simulink model "ObsvrCmptmtT"  (R2022b)
settings: Solver=FixedStepDiscrete  StartTime=0.0  StopTime=10.0  FixedStep=1  RelTol=1e-3
settings Model: Creator=blue.ge  LastModifiedBy=blue.ge
settings DataIOCC: SaveFormat=StructureWithTime
settings OptimizationCC: DefaultParameterBehavior=Inlined  EnhancedBackFolding=on  PassReuseOutputArgsAs=Individual arguments  ZeroExternalMemoryAtStartup=off  ZeroInternalMemoryAtStartup=off  OptimizeBlockOrder=speed  DifferentSizesBufferReuse=on  OptimizationCustomize=off  EfficientTunableParamExpr=on
settings DebuggingCC: ParameterTunabilityLossMsg=error
settings ModelReferenceCC: ModelReferenceNumInstancesAllowed=Single
settings SFSimCC: MATLABDynamicMemAlloc=off
settings RTWCC: Description=AUTOSAR  SystemTargetFile=autosar.tlc  GenCodeOnly=on  TemplateMakefile=ert_default_tmf
settings CodeAppCC: ForceParamTrailComments=on  IgnoreCustomStorageClasses=off  OperatorAnnotations=on  SimulinkDataObjDesc=on  SFDataObjDesc=on  InsertBlockDesc=on
settings STFCustomTargetCC: TargetLangStandard=C99 (ISO)  MultiwordLength=256  ParMdlRefBuildCompliant=on  CompOptLevelCompliant=on  IncludeMdlTerminateFcn=off  CombineOutputUpdateFcns=on  SuppressErrorStatus=on  ERTCustomFileBanners=on  SupportNonFinite=off  SupportComplex=off  SupportContinuousTime=off  SupportNonInlinedSFcns=off  RemoveResetFunc=on  MATLABClassNameForMDSCustomization=Simulink.SoftwareTarget.ERTCustomization  AutosarCompliant=on  UseToolchainInfoCompliant=on  LookupTableObjectStructAxisOrder=2,1,3,4,...  LUTObjectStructOrderEvenSpacing=Size,Table,Breakpoints  SystemTargetFile=autosar.tlc
settings ERTTargetCC: TargetLangStandard=C99 (ISO)  ConcurrentExecutionCompliant=off  IncludeMdlTerminateFcn=off  SuppressErrorStatus=on  SupportNonFinite=off  SupportComplex=off  ModelStepFunctionPrototypeControlCompliant=off  CPPClassGenCompliant=off  AutosarCompliant=on  LookupTableObjectStructAxisOrder=2,1,3,4,...  LUTObjectStructOrderEvenSpacing=Size,Table,Breakpoints  InlinedParameterPlacement=NonHierarchical  ERTCustomFileTemplate=example_file_process.tlc

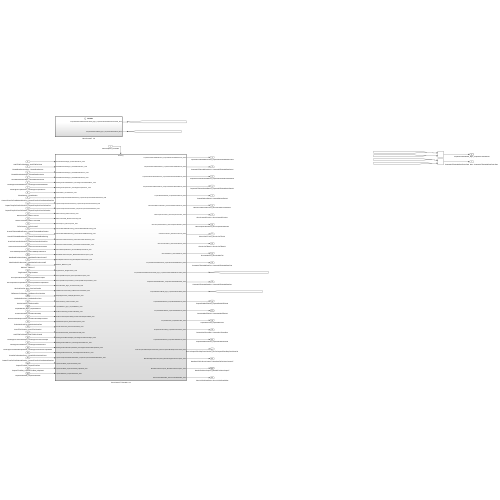
[thumbnail: Simulink's stored preview of the top-level diagram]
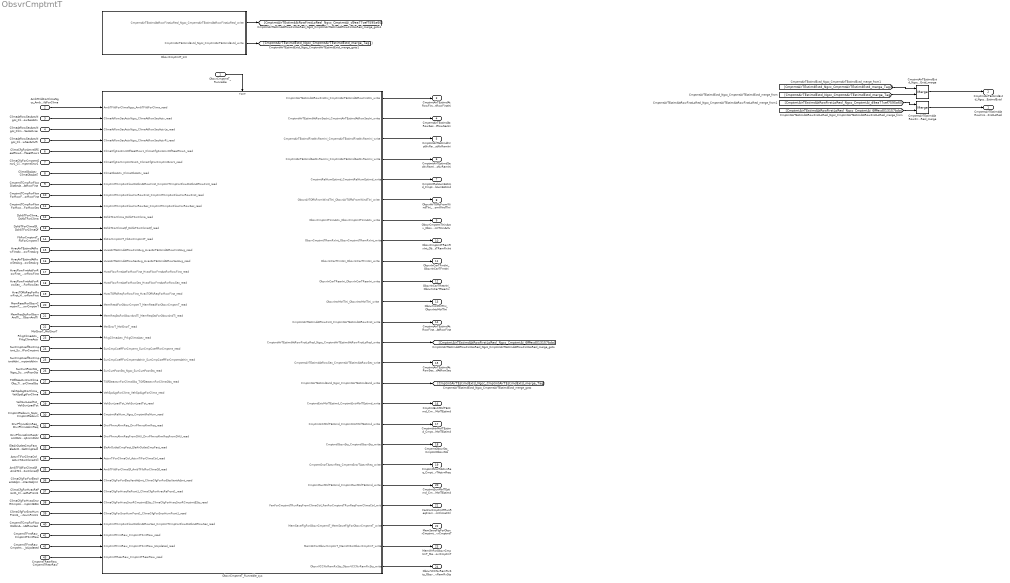
== system ObsvrCmptmtT ==
  ObsvrCmptmtT_Runnable : Inport  SampleTime=1  OutputFunctionCall=on
  AmbTFildForClimaNgcc_AmbTFildForClima : Inport  Port=2  OutDataTypeStr=T30  PortDimensions=1
  ClimaAtRowSecActvNgcc_ClimaAtRowSecActv : Inport  Port=3  OutDataTypeStr=Boolean  PortDimensions=1
  ClimaAtRowSecActvNgcc_ClimaAtRowSecActvLe : Inport  Port=4  OutDataTypeStr=Boolean  PortDimensions=1
  ClimaAtRowSecActvNgcc_ClimaAtRowSecActvRi : Inport  Port=5  OutDataTypeStr=Boolean  PortDimensions=1
  ClimaCfgForAmntOfSeatRow1_ClimaCfgForAmntOfSeatRow1 : Inport  Port=6  OutDataTypeStr=Enum: ClimaCfgForAmntOfSeatRow1  PortDimensions=1
  ClimaCfgForCmptmtSnsr1_ClimaCfgForCmptmtSnsr1 : Inport  Port=7  OutDataTypeStr=Enum: ClimaCfgForCmptmtSnsr1  PortDimensions=1
  ClimaObsActv_ClimaObsActv : Inport  Port=8  OutDataTypeStr=Boolean  PortDimensions=1
  CmptmtTCmpForFlowDistbnAtRowFirst_CmptmtTCmpForFlowDistbnAtRowFirst : Inport  Port=9  OutDataTypeStr=Fac30  PortDimensions=1
  CmptmtTCmpForFlowForRowFirst_CmptmtTCmpForFlowForRowFirst : Inport  Port=10  OutDataTypeStr=Fac30  PortDimensions=1
  CmptmtTCmpForFlowForRowSec_CmptmtTCmpForFlowForRowSec : Inport  Port=11  OutDataTypeStr=Fac30  PortDimensions=1
  DshbTForClima_DshbTForClima : Inport  Port=12  OutDataTypeStr=T30  PortDimensions=1
  DshbTForClimaQf_DshbTForClimaQf : Inport  Port=13  OutDataTypeStr=Enum: DshbTForClimaQf  PortDimensions=1
  FbForCmptmtT_FbForCmptmtT : Inport  Port=14  OutDataTypeStr=T30  PortDimensions=1
  HvacAirTEstimdAtRowFirstAvg_HvacAirTEstimdAtRowFirstAvg : Inport  Port=15  OutDataTypeStr=T30  PortDimensions=1
  HvacAirTEstimdAtRowSecAvg_HvacAirTEstimdAtRowSecAvg : Inport  Port=16  OutDataTypeStr=T30  PortDimensions=1
  HvacFlowFrntActForRowFirst_HvacFlowFrntActForRowFirst : Inport  Port=17  OutDataTypeStr=AirFlow30  PortDimensions=1
  HvacFlowFrntActForRowSec_HvacFlowFrntActForRowSec : Inport  Port=18  OutDataTypeStr=AirFlow30  PortDimensions=1
  HvacTOffsReqForRowFirst_HvacTOffsReqForRowFirst : Inport  Port=19  OutDataTypeStr=T30  PortDimensions=1
  MemReadForObsvrCmptmT_MemReadForObsvrCmptmT : Inport  Port=20  OutDataTypeStr=Bus: MemRecForObsvrCmptmT  PortDimensions=1  BusOutputAsStruct=on
  MemReqStsForObsvrAndTi_MemReqStsForObsvrAndTi : Inport  Port=21  OutDataTypeStr=Enum: MemReqSts  PortDimensions=1
  MtrlSnsrT_MtrlSnsrT : Inport  Port=22  OutDataTypeStr=Bus: MtrlSnsrT  PortDimensions=1  BusOutputAsStruct=on
  PrkgClimaActv_PrkgClimaActv : Inport  Port=23  OutDataTypeStr=Enum: PrkgClimaActv  PortDimensions=1
  SunCmpCoeffForCmptmt_SunCmpCoeffForCmptmt : Inport  Port=24  OutDataTypeStr=Fac30  PortDimensions=1
  SunCmpCoeffForCmptmtAtInin_SunCmpCoeffForCmptmtAtInin : Inport  Port=25  OutDataTypeStr=FacForSunCmpInin  PortDimensions=1
  SunCurtPosnSts_Ngcc_SunCurtPosnSts : Inport  Port=26  OutDataTypeStr=Enum: WinAndRoofAndCurtPosnTyp  PortDimensions=1
  TiOfDeactvnForClimaObs_TiOfDeactvnForClimaObs : Inport  Port=27  OutDataTypeStr=Ti33  PortDimensions=1
  VehSpdLgtForClima_VehSpdLgtForClima : Inport  Port=28  OutDataTypeStr=Bus: VehSpdLgtForClima  PortDimensions=1  BusOutputAsStruct=on
  VehSunLoadTot_VehSunLoadTot : Inport  Port=29  OutDataTypeStr=SunLoad30  PortDimensions=1
  CmptmtRelHum_Ngcc_CmptmtRelHum : Inport  Port=30  OutDataTypeStr=Bus: CmptmRelHum  PortDimensions=1  BusOutputAsStruct=on
  DrvrPfmncAlrmReq_DrvrPfmncAlrmReq : Inport  Port=31  OutDataTypeStr=Enum: DrvrPfmncWarnReq  PortDimensions=1
  DrvrPfmncAlrmReqFromDHU_DrvrPfmncAlrmReqFromDHU : Inport  Port=32  OutDataTypeStr=Enum: DrvrPfmncWarnReq  PortDimensions=1
  EleAirOutletCmpFact_EleAirOutletCmpFact : Inport  Port=33  OutDataTypeStr=VCCFac30  PortDimensions=1
  ActvnTiForClimaCtrl_ActvnTiForClimaCtrl : Inport  Port=34  OutDataTypeStr=Ti33  PortDimensions=1
  AmbTFildForClimaQf_AmbTFildForClimaQf : Inport  Port=35  OutDataTypeStr=Enum: GenQf1  PortDimensions=1
  ClimaCfgForForElecVentAdjmt_ClimaCfgForForElecVentAdjmt : Inport  Port=36  OutDataTypeStr=Boolean  PortDimensions=1
  ClimaCfgForHvacRePrsnt1_ClimaCfgForHvacRePrsnt1 : Inport  Port=37  OutDataTypeStr=Enum: CarCfgForHvacRe  PortDimensions=1
  ClimaCfgForHvacSnsrRCmptmtESts_ClimaCfgForHvacSnsrRCmptmtESts : Inport  Port=38  OutDataTypeStr=Enum: ClimaCfgForHvacSnsrRCmptmtESts  PortDimensions=1
  ClimaCfgForSnsrHumPrsnt1_ClimaCfgForSnsrHumPrsnt1 : Inport  Port=39  OutDataTypeStr=Boolean  PortDimensions=1
  CmptmtTCmpForFlowDistbnAtRowSec_CmptmtTCmpForFlowDistbnAtRowSec : Inport  Port=40  OutDataTypeStr=Fac30  PortDimensions=1
  CmptmtTFrntRaw_CmptmtTFrntRaw : Inport  Port=41  OutDataTypeStr=Bus: CmptmtTFrnt  PortDimensions=1  BusOutputAsStruct=on
  CmptmtTFrntRaw_CmptmtTFrntRaw_IsUpdated : Inport  Port=42  OutDataTypeStr=boolean  PortDimensions=1
  CmptmtTRearRaw_CmptmtTRearRaw : Inport  Port=43  OutDataTypeStr=Bus: CmptmtTFrnt  PortDimensions=1  BusOutputAsStruct=on
  CmptmtAirTEstimdAtRowFirstLoResl_Ngcc_CmptmtAirTEstimdAtRowFirstLoResl_merge : Merge
  CmptmtAirTEstimdExtd_Ngcc_CmptmtAirTEstimdExtd_merge : Merge
  ObsvrCmptmtT_Init : Subsystem
  ObsvrCmptmtT_Runnable_sys : Subsystem
  CmptmtAirTEstimdAtRowFirstLoResl_Ngcc_CmptmtAirTEstimdAtRowFirstLoResl : Outport  OutMin=-60  OutMax=125  OutDataTypeStr=TForCmptmt  PortDimensions=1
  CmptmtAirTEstimdExtd_Ngcc_CmptmtAirTEstimdExtd : Outport  Port=2  OutDataTypeStr=Bus: CmptmtAirTEstimdExtd  PortDimensions=1  BusOutputAsStruct=on
  CmptmtAirTEstimdAtRowFirstIni_CmptmtAirTEstimdAtRowFirstIni : Outport  Port=3  OutDataTypeStr=SInt8  PortDimensions=1
  CmptmtAirTEstimdAtRowSecIni_CmptmtAirTEstimdAtRowSecIni : Outport  Port=4  OutDataTypeStr=SInt8  PortDimensions=1
  CmptmtAirTEstimdFirstNvRamIni_CmptmtAirTEstimdFirstNvRamIni : Outport  Port=5  OutDataTypeStr=SInt8  PortDimensions=1
  CmptmtAirTEstimdSecNvRamIni_CmptmtAirTEstimdSecNvRamIni : Outport  Port=6  OutDataTypeStr=SInt8  PortDimensions=1
  CmptmtRelHumEstimd_CmptmtRelHumEstimd : Outport  Port=7  OutDataTypeStr=Perc7  PortDimensions=1
  ObsvrAirTOffsFromWindTIni_ObsvrAirTOffsFromWindTIni : Outport  Port=8  OutDataTypeStr=SInt8  PortDimensions=1
  ObsvrCmptmtTIninActv_ObsvrCmptmtTIninActv : Outport  Port=9  OutDataTypeStr=UInt8  PortDimensions=1
  ObsvrCmptmtTRamRxInt_ObsvrCmptmtTRamRxInt : Outport  Port=10  OutDataTypeStr=Enum: VCCNvRamRxSts  PortDimensions=1
  ObsvrInCarTFrntIni_ObsvrInCarTFrntIni : Outport  Port=11  OutDataTypeStr=SInt8  PortDimensions=1
  ObsvrInCarTRearIni_ObsvrInCarTRearIni : Outport  Port=12  OutDataTypeStr=SInt8  PortDimensions=1
  ObsvrIntrMtrlTIni_ObsvrIntrMtrlTIni : Outport  Port=13  OutDataTypeStr=SInt8  PortDimensions=1
  CmptmtAirTEstimdAtRowFirst_CmptmtAirTEstimdAtRowFirst : Outport  Port=14  OutDataTypeStr=T30  PortDimensions=1
  CmptmtAirTEstimdAtRowSec_CmptmtAirTEstimdAtRowSec : Outport  Port=15  OutDataTypeStr=T30  PortDimensions=1
  CmptmtExtrMtrlTEstimd_CmptmtExtrMtrlTEstimd : Outport  Port=16  OutDataTypeStr=T30  PortDimensions=1
  CmptmtIntrMtrlTEstimd_CmptmtIntrMtrlTEstimd : Outport  Port=17  OutDataTypeStr=Bus: MtrlSnsrT  PortDimensions=1  BusOutputAsStruct=on
  CmptmtObsvrSts_CmptmtObsvrSts : Outport  Port=18  OutDataTypeStr=Enum: ObsvrStsForCmptmt  PortDimensions=1
  CmptmtSnsrTActvnReq_CmptmtSnsrTActvnReq : Outport  Port=19  OutDataTypeStr=Enum: OnOff1  PortDimensions=1
  CmptmtSunMtrlTEstimd_CmptmtSunMtrlTEstimd : Outport  Port=20  OutDataTypeStr=T30  PortDimensions=1
  FanForCmptmtTRunReqFromClimaCtrl_FanForCmptmtTRunReqFromClimaCtrl : Outport  Port=21  OutDataTypeStr=Enum: OnOff1  PortDimensions=1
  MemSaveFlgForObsvrCmptmtT_MemSaveFlgForObsvrCmptmtT : Outport  Port=22  OutDataTypeStr=Boolean  PortDimensions=1
  MemWrForObsvrCmptmT_MemWrForObsvrCmptmT : Outport  Port=23  OutDataTypeStr=Bus: MemRecForObsvrCmptmT  PortDimensions=1  BusOutputAsStruct=on
  ObsvrVCCNvRamRxSts_ObsvrVCCNvRamRxSts : Outport  Port=24  OutDataTypeStr=Enum: VCCNvRamRxSts  PortDimensions=1
  wires:
  AmbTFildForClimaNgcc_AmbTFildForClima/1 -> ObsvrCmptmtT_Runnable_sys/1(AmbTFildForClimaNgcc_AmbTFildForClima_read)
  ClimaAtRowSecActvNgcc_ClimaAtRowSecActv/1 -> ObsvrCmptmtT_Runnable_sys/2(ClimaAtRowSecActvNgcc_ClimaAtRowSecActv_read)
  ClimaAtRowSecActvNgcc_ClimaAtRowSecActvLe/1 -> ObsvrCmptmtT_Runnable_sys/3(ClimaAtRowSecActvNgcc_ClimaAtRowSecActvLe_read)
  ClimaAtRowSecActvNgcc_ClimaAtRowSecActvRi/1 -> ObsvrCmptmtT_Runnable_sys/4(ClimaAtRowSecActvNgcc_ClimaAtRowSecActvRi_read)
  ClimaCfgForAmntOfSeatRow1_ClimaCfgForAmntOfSeatRow1/1 -> ObsvrCmptmtT_Runnable_sys/5(ClimaCfgForAmntOfSeatRow1_ClimaCfgForAmntOfSeatRow1_read)
  ClimaCfgForCmptmtSnsr1_ClimaCfgForCmptmtSnsr1/1 -> ObsvrCmptmtT_Runnable_sys/6(ClimaCfgForCmptmtSnsr1_ClimaCfgForCmptmtSnsr1_read)
  ClimaObsActv_ClimaObsActv/1 -> ObsvrCmptmtT_Runnable_sys/7(ClimaObsActv_ClimaObsActv_read)
  CmptmtTCmpForFlowDistbnAtRowFirst_CmptmtTCmpForFlowDistbnAtRowFirst/1 -> ObsvrCmptmtT_Runnable_sys/8(CmptmtTCmpForFlowDistbnAtRowFirst_CmptmtTCmpForFlowDistbnAtRowFirst_read)
  CmptmtTCmpForFlowForRowFirst_CmptmtTCmpForFlowForRowFirst/1 -> ObsvrCmptmtT_Runnable_sys/9(CmptmtTCmpForFlowForRowFirst_CmptmtTCmpForFlowForRowFirst_read)
  CmptmtTCmpForFlowForRowSec_CmptmtTCmpForFlowForRowSec/1 -> ObsvrCmptmtT_Runnable_sys/10(CmptmtTCmpForFlowForRowSec_CmptmtTCmpForFlowForRowSec_read)
  DshbTForClima_DshbTForClima/1 -> ObsvrCmptmtT_Runnable_sys/11(DshbTForClima_DshbTForClima_read)
  DshbTForClimaQf_DshbTForClimaQf/1 -> ObsvrCmptmtT_Runnable_sys/12(DshbTForClimaQf_DshbTForClimaQf_read)
  FbForCmptmtT_FbForCmptmtT/1 -> ObsvrCmptmtT_Runnable_sys/13(FbForCmptmtT_FbForCmptmtT_read)
  HvacAirTEstimdAtRowFirstAvg_HvacAirTEstimdAtRowFirstAvg/1 -> ObsvrCmptmtT_Runnable_sys/14(HvacAirTEstimdAtRowFirstAvg_HvacAirTEstimdAtRowFirstAvg_read)
  HvacAirTEstimdAtRowSecAvg_HvacAirTEstimdAtRowSecAvg/1 -> ObsvrCmptmtT_Runnable_sys/15(HvacAirTEstimdAtRowSecAvg_HvacAirTEstimdAtRowSecAvg_read)
  HvacFlowFrntActForRowFirst_HvacFlowFrntActForRowFirst/1 -> ObsvrCmptmtT_Runnable_sys/16(HvacFlowFrntActForRowFirst_HvacFlowFrntActForRowFirst_read)
  HvacFlowFrntActForRowSec_HvacFlowFrntActForRowSec/1 -> ObsvrCmptmtT_Runnable_sys/17(HvacFlowFrntActForRowSec_HvacFlowFrntActForRowSec_read)
  HvacTOffsReqForRowFirst_HvacTOffsReqForRowFirst/1 -> ObsvrCmptmtT_Runnable_sys/18(HvacTOffsReqForRowFirst_HvacTOffsReqForRowFirst_read)
  MemReadForObsvrCmptmT_MemReadForObsvrCmptmT/1 -> ObsvrCmptmtT_Runnable_sys/19(MemReadForObsvrCmptmT_MemReadForObsvrCmptmT_read)
  MemReqStsForObsvrAndTi_MemReqStsForObsvrAndTi/1 -> ObsvrCmptmtT_Runnable_sys/20(MemReqStsForObsvrAndTi_MemReqStsForObsvrAndTi_read)
  MtrlSnsrT_MtrlSnsrT/1 -> ObsvrCmptmtT_Runnable_sys/21(MtrlSnsrT_MtrlSnsrT_read)
  PrkgClimaActv_PrkgClimaActv/1 -> ObsvrCmptmtT_Runnable_sys/22(PrkgClimaActv_PrkgClimaActv_read)
  SunCmpCoeffForCmptmt_SunCmpCoeffForCmptmt/1 -> ObsvrCmptmtT_Runnable_sys/23(SunCmpCoeffForCmptmt_SunCmpCoeffForCmptmt_read)
  SunCmpCoeffForCmptmtAtInin_SunCmpCoeffForCmptmtAtInin/1 -> ObsvrCmptmtT_Runnable_sys/24(SunCmpCoeffForCmptmtAtInin_SunCmpCoeffForCmptmtAtInin_read)
  SunCurtPosnSts_Ngcc_SunCurtPosnSts/1 -> ObsvrCmptmtT_Runnable_sys/25(SunCurtPosnSts_Ngcc_SunCurtPosnSts_read)
  TiOfDeactvnForClimaObs_TiOfDeactvnForClimaObs/1 -> ObsvrCmptmtT_Runnable_sys/26(TiOfDeactvnForClimaObs_TiOfDeactvnForClimaObs_read)
  VehSpdLgtForClima_VehSpdLgtForClima/1 -> ObsvrCmptmtT_Runnable_sys/27(VehSpdLgtForClima_VehSpdLgtForClima_read)
  VehSunLoadTot_VehSunLoadTot/1 -> ObsvrCmptmtT_Runnable_sys/28(VehSunLoadTot_VehSunLoadTot_read)
  CmptmtRelHum_Ngcc_CmptmtRelHum/1 -> ObsvrCmptmtT_Runnable_sys/29(CmptmtRelHum_Ngcc_CmptmtRelHum_read)
  DrvrPfmncAlrmReq_DrvrPfmncAlrmReq/1 -> ObsvrCmptmtT_Runnable_sys/30(DrvrPfmncAlrmReq_DrvrPfmncAlrmReq_read)
  DrvrPfmncAlrmReqFromDHU_DrvrPfmncAlrmReqFromDHU/1 -> ObsvrCmptmtT_Runnable_sys/31(DrvrPfmncAlrmReqFromDHU_DrvrPfmncAlrmReqFromDHU_read)
  EleAirOutletCmpFact_EleAirOutletCmpFact/1 -> ObsvrCmptmtT_Runnable_sys/32(EleAirOutletCmpFact_EleAirOutletCmpFact_read)
  ActvnTiForClimaCtrl_ActvnTiForClimaCtrl/1 -> ObsvrCmptmtT_Runnable_sys/33(ActvnTiForClimaCtrl_ActvnTiForClimaCtrl_read)
  AmbTFildForClimaQf_AmbTFildForClimaQf/1 -> ObsvrCmptmtT_Runnable_sys/34(AmbTFildForClimaQf_AmbTFildForClimaQf_read)
  ClimaCfgForForElecVentAdjmt_ClimaCfgForForElecVentAdjmt/1 -> ObsvrCmptmtT_Runnable_sys/35(ClimaCfgForForElecVentAdjmt_ClimaCfgForForElecVentAdjmt_read)
  ClimaCfgForHvacRePrsnt1_ClimaCfgForHvacRePrsnt1/1 -> ObsvrCmptmtT_Runnable_sys/36(ClimaCfgForHvacRePrsnt1_ClimaCfgForHvacRePrsnt1_read)
  ClimaCfgForHvacSnsrRCmptmtESts_ClimaCfgForHvacSnsrRCmptmtESts/1 -> ObsvrCmptmtT_Runnable_sys/37(ClimaCfgForHvacSnsrRCmptmtESts_ClimaCfgForHvacSnsrRCmptmtESts_read)
  ClimaCfgForSnsrHumPrsnt1_ClimaCfgForSnsrHumPrsnt1/1 -> ObsvrCmptmtT_Runnable_sys/38(ClimaCfgForSnsrHumPrsnt1_ClimaCfgForSnsrHumPrsnt1_read)
  CmptmtTCmpForFlowDistbnAtRowSec_CmptmtTCmpForFlowDistbnAtRowSec/1 -> ObsvrCmptmtT_Runnable_sys/39(CmptmtTCmpForFlowDistbnAtRowSec_CmptmtTCmpForFlowDistbnAtRowSec_read)
  CmptmtTFrntRaw_CmptmtTFrntRaw/1 -> ObsvrCmptmtT_Runnable_sys/40(CmptmtTFrntRaw_CmptmtTFrntRaw_read)
  CmptmtTFrntRaw_CmptmtTFrntRaw_IsUpdated/1 -> ObsvrCmptmtT_Runnable_sys/41(CmptmtTFrntRaw_CmptmtTFrntRaw_IsUpdated_read)
  CmptmtTRearRaw_CmptmtTRearRaw/1 -> ObsvrCmptmtT_Runnable_sys/42(CmptmtTRearRaw_CmptmtTRearRaw_read)
  ObsvrCmptmtT_Runnable_sys/1(CmptmtAirTEstimdAtRowFirstIni_CmptmtAirTEstimdAtRowFirstIni_write) -> CmptmtAirTEstimdAtRowFirstIni_CmptmtAirTEstimdAtRowFirstIni/1
  ObsvrCmptmtT_Runnable_sys/2(CmptmtAirTEstimdAtRowSecIni_CmptmtAirTEstimdAtRowSecIni_write) -> CmptmtAirTEstimdAtRowSecIni_CmptmtAirTEstimdAtRowSecIni/1
  ObsvrCmptmtT_Runnable_sys/3(CmptmtAirTEstimdFirstNvRamIni_CmptmtAirTEstimdFirstNvRamIni_write) -> CmptmtAirTEstimdFirstNvRamIni_CmptmtAirTEstimdFirstNvRamIni/1
  ObsvrCmptmtT_Runnable_sys/4(CmptmtAirTEstimdSecNvRamIni_CmptmtAirTEstimdSecNvRamIni_write) -> CmptmtAirTEstimdSecNvRamIni_CmptmtAirTEstimdSecNvRamIni/1
  ObsvrCmptmtT_Runnable_sys/5(CmptmtRelHumEstimd_CmptmtRelHumEstimd_write) -> CmptmtRelHumEstimd_CmptmtRelHumEstimd/1
  ObsvrCmptmtT_Runnable_sys/6(ObsvrAirTOffsFromWindTIni_ObsvrAirTOffsFromWindTIni_write) -> ObsvrAirTOffsFromWindTIni_ObsvrAirTOffsFromWindTIni/1
  ObsvrCmptmtT_Runnable_sys/7(ObsvrCmptmtTIninActv_ObsvrCmptmtTIninActv_write) -> ObsvrCmptmtTIninActv_ObsvrCmptmtTIninActv/1
  ObsvrCmptmtT_Runnable_sys/8(ObsvrCmptmtTRamRxInt_ObsvrCmptmtTRamRxInt_write) -> ObsvrCmptmtTRamRxInt_ObsvrCmptmtTRamRxInt/1
  ObsvrCmptmtT_Runnable_sys/9(ObsvrInCarTFrntIni_ObsvrInCarTFrntIni_write) -> ObsvrInCarTFrntIni_ObsvrInCarTFrntIni/1
  ObsvrCmptmtT_Runnable_sys/10(ObsvrInCarTRearIni_ObsvrInCarTRearIni_write) -> ObsvrInCarTRearIni_ObsvrInCarTRearIni/1
  ObsvrCmptmtT_Runnable_sys/11(ObsvrIntrMtrlTIni_ObsvrIntrMtrlTIni_write) -> ObsvrIntrMtrlTIni_ObsvrIntrMtrlTIni/1
  ObsvrCmptmtT_Runnable_sys/12(CmptmtAirTEstimdAtRowFirst_CmptmtAirTEstimdAtRowFirst_write) -> CmptmtAirTEstimdAtRowFirst_CmptmtAirTEstimdAtRowFirst/1
  CmptmtAirTEstimdAtRowFirstLoResl_Ngcc_CmptmtAirTEstimdAtRowFirstLoResl_merge/1 -> CmptmtAirTEstimdAtRowFirstLoResl_Ngcc_CmptmtAirTEstimdAtRowFirstLoResl/1
  ObsvrCmptmtT_Runnable_sys/14(CmptmtAirTEstimdAtRowSec_CmptmtAirTEstimdAtRowSec_write) -> CmptmtAirTEstimdAtRowSec_CmptmtAirTEstimdAtRowSec/1
  CmptmtAirTEstimdExtd_Ngcc_CmptmtAirTEstimdExtd_merge/1 -> CmptmtAirTEstimdExtd_Ngcc_CmptmtAirTEstimdExtd/1
  ObsvrCmptmtT_Runnable_sys/16(CmptmtExtrMtrlTEstimd_CmptmtExtrMtrlTEstimd_write) -> CmptmtExtrMtrlTEstimd_CmptmtExtrMtrlTEstimd/1
  ObsvrCmptmtT_Runnable_sys/17(CmptmtIntrMtrlTEstimd_CmptmtIntrMtrlTEstimd_write) -> CmptmtIntrMtrlTEstimd_CmptmtIntrMtrlTEstimd/1
  ObsvrCmptmtT_Runnable_sys/18(CmptmtObsvrSts_CmptmtObsvrSts_write) -> CmptmtObsvrSts_CmptmtObsvrSts/1
  ObsvrCmptmtT_Runnable_sys/19(CmptmtSnsrTActvnReq_CmptmtSnsrTActvnReq_write) -> CmptmtSnsrTActvnReq_CmptmtSnsrTActvnReq/1
  ObsvrCmptmtT_Runnable_sys/20(CmptmtSunMtrlTEstimd_CmptmtSunMtrlTEstimd_write) -> CmptmtSunMtrlTEstimd_CmptmtSunMtrlTEstimd/1
  ObsvrCmptmtT_Runnable_sys/21(FanForCmptmtTRunReqFromClimaCtrl_FanForCmptmtTRunReqFromClimaCtrl_write) -> FanForCmptmtTRunReqFromClimaCtrl_FanForCmptmtTRunReqFromClimaCtrl/1
  ObsvrCmptmtT_Runnable_sys/22(MemSaveFlgForObsvrCmptmtT_MemSaveFlgForObsvrCmptmtT_write) -> MemSaveFlgForObsvrCmptmtT_MemSaveFlgForObsvrCmptmtT/1
  ObsvrCmptmtT_Runnable_sys/23(MemWrForObsvrCmptmT_MemWrForObsvrCmptmT_write) -> MemWrForObsvrCmptmT_MemWrForObsvrCmptmT/1
  ObsvrCmptmtT_Runnable_sys/24(ObsvrVCCNvRamRxSts_ObsvrVCCNvRamRxSts_write) -> ObsvrVCCNvRamRxSts_ObsvrVCCNvRamRxSts/1
  ObsvrCmptmtT_Runnable_sys/15(CmptmtAirTEstimdExtd_Ngcc_CmptmtAirTEstimdExtd_write) -> [CmptmtAirTEstimdExtd_Ngcc_CmptmtAirTEstimdExtd_merge_Tag]
  [CmptmtAirTEstimdExtd_Ngcc_CmptmtAirTEstimdExtd_merge_Tag] -> CmptmtAirTEstimdExtd_Ngcc_CmptmtAirTEstimdExtd_merge/2
  ObsvrCmptmtT_Init/2(CmptmtAirTEstimdExtd_Ngcc_CmptmtAirTEstimdExtd_write) -> [CmptmtAirTEstimdExtd_Ngcc_CmptmtAirTEstimdExtd_merge_Tag1]
  [CmptmtAirTEstimdExtd_Ngcc_CmptmtAirTEstimdExtd_merge_Tag1] -> CmptmtAirTEstimdExtd_Ngcc_CmptmtAirTEstimdExtd_merge/1
  ObsvrCmptmtT_Runnable_sys/13(CmptmtAirTEstimdAtRowFirstLoResl_Ngcc_CmptmtAirTEstimdAtRowFirstLoResl_write) -> [CmptmtAirTEstimdAtRowFirstLoResl_Ngcc_CmptmtAi_6fffecd013157bda]
  [CmptmtAirTEstimdAtRowFirstLoResl_Ngcc_CmptmtAi_6fffecd013157bda] -> CmptmtAirTEstimdAtRowFirstLoResl_Ngcc_CmptmtAirTEstimdAtRowFirstLoResl_merge/2
  ObsvrCmptmtT_Init/1(CmptmtAirTEstimdAtRowFirstLoResl_Ngcc_CmptmtAirTEstimdAtRowFirstLoResl_write) -> [CmptmtAirTEstimdAtRowFirstLoResl_Ngcc_CmptmtAi_d9ea77cef7595a60]
  [CmptmtAirTEstimdAtRowFirstLoResl_Ngcc_CmptmtAi_d9ea77cef7595a60] -> CmptmtAirTEstimdAtRowFirstLoResl_Ngcc_CmptmtAirTEstimdAtRowFirstLoResl_merge/1
  ObsvrCmptmtT_Runnable/1 -> ObsvrCmptmtT_Runnable_sys/Trigger

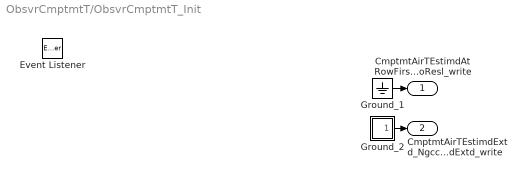
== system ObsvrCmptmtT/ObsvrCmptmtT_Init ==
  Event Listener : EventListener  EventName=reset
  Ground_1 : Ground
  Ground_2 : Subsystem
  CmptmtAirTEstimdAtRowFirstLoResl_Ngcc_CmptmtAirTEstimdAtRowFirstLoResl_write : Outport
  CmptmtAirTEstimdExtd_Ngcc_CmptmtAirTEstimdExtd_write : Outport  Port=2
  wires:
  Ground_1/1 -> CmptmtAirTEstimdAtRowFirstLoResl_Ngcc_CmptmtAirTEstimdAtRowFirstLoResl_write/1
  Ground_2/1(Out1) -> CmptmtAirTEstimdExtd_Ngcc_CmptmtAirTEstimdExtd_write/1

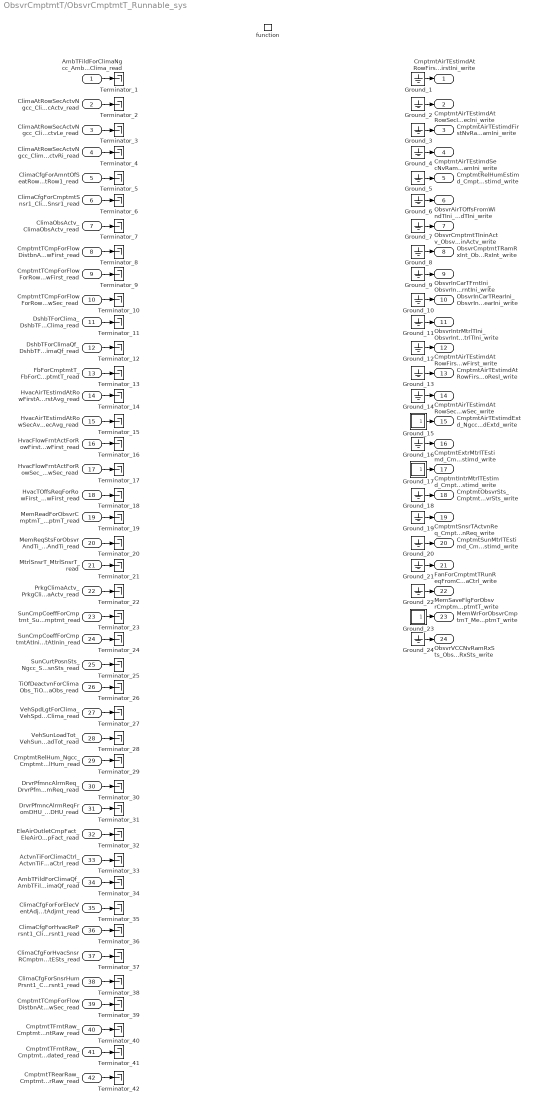
== system ObsvrCmptmtT/ObsvrCmptmtT_Runnable_sys ==
  AmbTFildForClimaNgcc_AmbTFildForClima_read : Inport
  ClimaAtRowSecActvNgcc_ClimaAtRowSecActv_read : Inport  Port=2
  ClimaAtRowSecActvNgcc_ClimaAtRowSecActvLe_read : Inport  Port=3
  ClimaAtRowSecActvNgcc_ClimaAtRowSecActvRi_read : Inport  Port=4
  ClimaCfgForAmntOfSeatRow1_ClimaCfgForAmntOfSeatRow1_read : Inport  Port=5
  ClimaCfgForCmptmtSnsr1_ClimaCfgForCmptmtSnsr1_read : Inport  Port=6
  ClimaObsActv_ClimaObsActv_read : Inport  Port=7
  CmptmtTCmpForFlowDistbnAtRowFirst_CmptmtTCmpForFlowDistbnAtRowFirst_read : Inport  Port=8
  CmptmtTCmpForFlowForRowFirst_CmptmtTCmpForFlowForRowFirst_read : Inport  Port=9
  CmptmtTCmpForFlowForRowSec_CmptmtTCmpForFlowForRowSec_read : Inport  Port=10
  DshbTForClima_DshbTForClima_read : Inport  Port=11
  DshbTForClimaQf_DshbTForClimaQf_read : Inport  Port=12
  FbForCmptmtT_FbForCmptmtT_read : Inport  Port=13
  HvacAirTEstimdAtRowFirstAvg_HvacAirTEstimdAtRowFirstAvg_read : Inport  Port=14
  HvacAirTEstimdAtRowSecAvg_HvacAirTEstimdAtRowSecAvg_read : Inport  Port=15
  HvacFlowFrntActForRowFirst_HvacFlowFrntActForRowFirst_read : Inport  Port=16
  HvacFlowFrntActForRowSec_HvacFlowFrntActForRowSec_read : Inport  Port=17
  HvacTOffsReqForRowFirst_HvacTOffsReqForRowFirst_read : Inport  Port=18
  MemReadForObsvrCmptmT_MemReadForObsvrCmptmT_read : Inport  Port=19
  MemReqStsForObsvrAndTi_MemReqStsForObsvrAndTi_read : Inport  Port=20
  MtrlSnsrT_MtrlSnsrT_read : Inport  Port=21
  PrkgClimaActv_PrkgClimaActv_read : Inport  Port=22
  SunCmpCoeffForCmptmt_SunCmpCoeffForCmptmt_read : Inport  Port=23
  SunCmpCoeffForCmptmtAtInin_SunCmpCoeffForCmptmtAtInin_read : Inport  Port=24
  SunCurtPosnSts_Ngcc_SunCurtPosnSts_read : Inport  Port=25
  TiOfDeactvnForClimaObs_TiOfDeactvnForClimaObs_read : Inport  Port=26
  VehSpdLgtForClima_VehSpdLgtForClima_read : Inport  Port=27
  VehSunLoadTot_VehSunLoadTot_read : Inport  Port=28
  CmptmtRelHum_Ngcc_CmptmtRelHum_read : Inport  Port=29
  DrvrPfmncAlrmReq_DrvrPfmncAlrmReq_read : Inport  Port=30
  DrvrPfmncAlrmReqFromDHU_DrvrPfmncAlrmReqFromDHU_read : Inport  Port=31
  EleAirOutletCmpFact_EleAirOutletCmpFact_read : Inport  Port=32
  ActvnTiForClimaCtrl_ActvnTiForClimaCtrl_read : Inport  Port=33
  AmbTFildForClimaQf_AmbTFildForClimaQf_read : Inport  Port=34
  ClimaCfgForForElecVentAdjmt_ClimaCfgForForElecVentAdjmt_read : Inport  Port=35
  ClimaCfgForHvacRePrsnt1_ClimaCfgForHvacRePrsnt1_read : Inport  Port=36
  ClimaCfgForHvacSnsrRCmptmtESts_ClimaCfgForHvacSnsrRCmptmtESts_read : Inport  Port=37
  ClimaCfgForSnsrHumPrsnt1_ClimaCfgForSnsrHumPrsnt1_read : Inport  Port=38
  CmptmtTCmpForFlowDistbnAtRowSec_CmptmtTCmpForFlowDistbnAtRowSec_read : Inport  Port=39
  CmptmtTFrntRaw_CmptmtTFrntRaw_read : Inport  Port=40
  CmptmtTFrntRaw_CmptmtTFrntRaw_IsUpdated_read : Inport  Port=41
  CmptmtTRearRaw_CmptmtTRearRaw_read : Inport  Port=42
  function : TriggerPort  TriggerType=function-call  VariantControl=(inherit)  SampleTimeType=periodic  SampleTime=-1
  Ground_{1..14,16,18..22,24} (21 alike) : Ground
  Ground_15 : Subsystem
  Ground_17 : Subsystem
  Ground_23 : Subsystem
  Terminator_{1..42} (42 alike) : Terminator
  CmptmtAirTEstimdAtRowFirstIni_CmptmtAirTEstimdAtRowFirstIni_write : Outport
      settings: EnsureOutportIsVirtual=on
  CmptmtAirTEstimdAtRowSecIni_CmptmtAirTEstimdAtRowSecIni_write : Outport  Port=2
      settings: EnsureOutportIsVirtual=on
  CmptmtAirTEstimdFirstNvRamIni_CmptmtAirTEstimdFirstNvRamIni_write : Outport  Port=3
      settings: EnsureOutportIsVirtual=on
  CmptmtAirTEstimdSecNvRamIni_CmptmtAirTEstimdSecNvRamIni_write : Outport  Port=4
      settings: EnsureOutportIsVirtual=on
  CmptmtRelHumEstimd_CmptmtRelHumEstimd_write : Outport  Port=5
      settings: EnsureOutportIsVirtual=on
  ObsvrAirTOffsFromWindTIni_ObsvrAirTOffsFromWindTIni_write : Outport  Port=6
      settings: EnsureOutportIsVirtual=on
  ObsvrCmptmtTIninActv_ObsvrCmptmtTIninActv_write : Outport  Port=7
      settings: EnsureOutportIsVirtual=on
  ObsvrCmptmtTRamRxInt_ObsvrCmptmtTRamRxInt_write : Outport  Port=8
      settings: EnsureOutportIsVirtual=on
  ObsvrInCarTFrntIni_ObsvrInCarTFrntIni_write : Outport  Port=9
      settings: EnsureOutportIsVirtual=on
  ObsvrInCarTRearIni_ObsvrInCarTRearIni_write : Outport  Port=10
      settings: EnsureOutportIsVirtual=on
  ObsvrIntrMtrlTIni_ObsvrIntrMtrlTIni_write : Outport  Port=11
      settings: EnsureOutportIsVirtual=on
  CmptmtAirTEstimdAtRowFirst_CmptmtAirTEstimdAtRowFirst_write : Outport  Port=12
      settings: EnsureOutportIsVirtual=on
  CmptmtAirTEstimdAtRowFirstLoResl_Ngcc_CmptmtAirTEstimdAtRowFirstLoResl_write : Outport  Port=13
      settings: EnsureOutportIsVirtual=on
  CmptmtAirTEstimdAtRowSec_CmptmtAirTEstimdAtRowSec_write : Outport  Port=14
      settings: EnsureOutportIsVirtual=on
  CmptmtAirTEstimdExtd_Ngcc_CmptmtAirTEstimdExtd_write : Outport  Port=15
      settings: EnsureOutportIsVirtual=on
  CmptmtExtrMtrlTEstimd_CmptmtExtrMtrlTEstimd_write : Outport  Port=16
      settings: EnsureOutportIsVirtual=on
  CmptmtIntrMtrlTEstimd_CmptmtIntrMtrlTEstimd_write : Outport  Port=17
      settings: EnsureOutportIsVirtual=on
  CmptmtObsvrSts_CmptmtObsvrSts_write : Outport  Port=18
      settings: EnsureOutportIsVirtual=on
  CmptmtSnsrTActvnReq_CmptmtSnsrTActvnReq_write : Outport  Port=19
      settings: EnsureOutportIsVirtual=on
  CmptmtSunMtrlTEstimd_CmptmtSunMtrlTEstimd_write : Outport  Port=20
      settings: EnsureOutportIsVirtual=on
  FanForCmptmtTRunReqFromClimaCtrl_FanForCmptmtTRunReqFromClimaCtrl_write : Outport  Port=21
      settings: EnsureOutportIsVirtual=on
  MemSaveFlgForObsvrCmptmtT_MemSaveFlgForObsvrCmptmtT_write : Outport  Port=22
      settings: EnsureOutportIsVirtual=on
  MemWrForObsvrCmptmT_MemWrForObsvrCmptmT_write : Outport  Port=23
      settings: EnsureOutportIsVirtual=on
  ObsvrVCCNvRamRxSts_ObsvrVCCNvRamRxSts_write : Outport  Port=24
      settings: EnsureOutportIsVirtual=on
  wires:
  AmbTFildForClimaNgcc_AmbTFildForClima_read/1 -> Terminator_1/1
  ClimaAtRowSecActvNgcc_ClimaAtRowSecActv_read/1 -> Terminator_2/1
  ClimaAtRowSecActvNgcc_ClimaAtRowSecActvLe_read/1 -> Terminator_3/1
  ClimaAtRowSecActvNgcc_ClimaAtRowSecActvRi_read/1 -> Terminator_4/1
  ClimaCfgForAmntOfSeatRow1_ClimaCfgForAmntOfSeatRow1_read/1 -> Terminator_5/1
  ClimaCfgForCmptmtSnsr1_ClimaCfgForCmptmtSnsr1_read/1 -> Terminator_6/1
  ClimaObsActv_ClimaObsActv_read/1 -> Terminator_7/1
  CmptmtTCmpForFlowDistbnAtRowFirst_CmptmtTCmpForFlowDistbnAtRowFirst_read/1 -> Terminator_8/1
  CmptmtTCmpForFlowForRowFirst_CmptmtTCmpForFlowForRowFirst_read/1 -> Terminator_9/1
  CmptmtTCmpForFlowForRowSec_CmptmtTCmpForFlowForRowSec_read/1 -> Terminator_10/1
  DshbTForClima_DshbTForClima_read/1 -> Terminator_11/1
  DshbTForClimaQf_DshbTForClimaQf_read/1 -> Terminator_12/1
  FbForCmptmtT_FbForCmptmtT_read/1 -> Terminator_13/1
  HvacAirTEstimdAtRowFirstAvg_HvacAirTEstimdAtRowFirstAvg_read/1 -> Terminator_14/1
  HvacAirTEstimdAtRowSecAvg_HvacAirTEstimdAtRowSecAvg_read/1 -> Terminator_15/1
  HvacFlowFrntActForRowFirst_HvacFlowFrntActForRowFirst_read/1 -> Terminator_16/1
  HvacFlowFrntActForRowSec_HvacFlowFrntActForRowSec_read/1 -> Terminator_17/1
  HvacTOffsReqForRowFirst_HvacTOffsReqForRowFirst_read/1 -> Terminator_18/1
  MemReadForObsvrCmptmT_MemReadForObsvrCmptmT_read/1 -> Terminator_19/1
  MemReqStsForObsvrAndTi_MemReqStsForObsvrAndTi_read/1 -> Terminator_20/1
  MtrlSnsrT_MtrlSnsrT_read/1 -> Terminator_21/1
  PrkgClimaActv_PrkgClimaActv_read/1 -> Terminator_22/1
  SunCmpCoeffForCmptmt_SunCmpCoeffForCmptmt_read/1 -> Terminator_23/1
  SunCmpCoeffForCmptmtAtInin_SunCmpCoeffForCmptmtAtInin_read/1 -> Terminator_24/1
  SunCurtPosnSts_Ngcc_SunCurtPosnSts_read/1 -> Terminator_25/1
  TiOfDeactvnForClimaObs_TiOfDeactvnForClimaObs_read/1 -> Terminator_26/1
  VehSpdLgtForClima_VehSpdLgtForClima_read/1 -> Terminator_27/1
  VehSunLoadTot_VehSunLoadTot_read/1 -> Terminator_28/1
  CmptmtRelHum_Ngcc_CmptmtRelHum_read/1 -> Terminator_29/1
  DrvrPfmncAlrmReq_DrvrPfmncAlrmReq_read/1 -> Terminator_30/1
  DrvrPfmncAlrmReqFromDHU_DrvrPfmncAlrmReqFromDHU_read/1 -> Terminator_31/1
  EleAirOutletCmpFact_EleAirOutletCmpFact_read/1 -> Terminator_32/1
  ActvnTiForClimaCtrl_ActvnTiForClimaCtrl_read/1 -> Terminator_33/1
  AmbTFildForClimaQf_AmbTFildForClimaQf_read/1 -> Terminator_34/1
  ClimaCfgForForElecVentAdjmt_ClimaCfgForForElecVentAdjmt_read/1 -> Terminator_35/1
  ClimaCfgForHvacRePrsnt1_ClimaCfgForHvacRePrsnt1_read/1 -> Terminator_36/1
  ClimaCfgForHvacSnsrRCmptmtESts_ClimaCfgForHvacSnsrRCmptmtESts_read/1 -> Terminator_37/1
  ClimaCfgForSnsrHumPrsnt1_ClimaCfgForSnsrHumPrsnt1_read/1 -> Terminator_38/1
  CmptmtTCmpForFlowDistbnAtRowSec_CmptmtTCmpForFlowDistbnAtRowSec_read/1 -> Terminator_39/1
  CmptmtTFrntRaw_CmptmtTFrntRaw_read/1 -> Terminator_40/1
  CmptmtTFrntRaw_CmptmtTFrntRaw_IsUpdated_read/1 -> Terminator_41/1
  CmptmtTRearRaw_CmptmtTRearRaw_read/1 -> Terminator_42/1
  Ground_1/1 -> CmptmtAirTEstimdAtRowFirstIni_CmptmtAirTEstimdAtRowFirstIni_write/1
  Ground_2/1 -> CmptmtAirTEstimdAtRowSecIni_CmptmtAirTEstimdAtRowSecIni_write/1
  Ground_3/1 -> CmptmtAirTEstimdFirstNvRamIni_CmptmtAirTEstimdFirstNvRamIni_write/1
  Ground_4/1 -> CmptmtAirTEstimdSecNvRamIni_CmptmtAirTEstimdSecNvRamIni_write/1
  Ground_5/1 -> CmptmtRelHumEstimd_CmptmtRelHumEstimd_write/1
  Ground_6/1 -> ObsvrAirTOffsFromWindTIni_ObsvrAirTOffsFromWindTIni_write/1
  Ground_7/1 -> ObsvrCmptmtTIninActv_ObsvrCmptmtTIninActv_write/1
  Ground_8/1 -> ObsvrCmptmtTRamRxInt_ObsvrCmptmtTRamRxInt_write/1
  Ground_9/1 -> ObsvrInCarTFrntIni_ObsvrInCarTFrntIni_write/1
  Ground_10/1 -> ObsvrInCarTRearIni_ObsvrInCarTRearIni_write/1
  Ground_11/1 -> ObsvrIntrMtrlTIni_ObsvrIntrMtrlTIni_write/1
  Ground_12/1 -> CmptmtAirTEstimdAtRowFirst_CmptmtAirTEstimdAtRowFirst_write/1
  Ground_13/1 -> CmptmtAirTEstimdAtRowFirstLoResl_Ngcc_CmptmtAirTEstimdAtRowFirstLoResl_write/1
  Ground_14/1 -> CmptmtAirTEstimdAtRowSec_CmptmtAirTEstimdAtRowSec_write/1
  Ground_15/1(Out1) -> CmptmtAirTEstimdExtd_Ngcc_CmptmtAirTEstimdExtd_write/1
  Ground_16/1 -> CmptmtExtrMtrlTEstimd_CmptmtExtrMtrlTEstimd_write/1
  Ground_17/1(Out1) -> CmptmtIntrMtrlTEstimd_CmptmtIntrMtrlTEstimd_write/1
  Ground_18/1 -> CmptmtObsvrSts_CmptmtObsvrSts_write/1
  Ground_19/1 -> CmptmtSnsrTActvnReq_CmptmtSnsrTActvnReq_write/1
  Ground_20/1 -> CmptmtSunMtrlTEstimd_CmptmtSunMtrlTEstimd_write/1
  Ground_21/1 -> FanForCmptmtTRunReqFromClimaCtrl_FanForCmptmtTRunReqFromClimaCtrl_write/1
  Ground_22/1 -> MemSaveFlgForObsvrCmptmtT_MemSaveFlgForObsvrCmptmtT_write/1
  Ground_23/1(Out1) -> MemWrForObsvrCmptmT_MemWrForObsvrCmptmT_write/1
  Ground_24/1 -> ObsvrVCCNvRamRxSts_ObsvrVCCNvRamRxSts_write/1

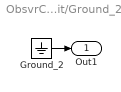
== system ObsvrCmptmtT/ObsvrCmptmtT_Init/Ground_2 ==
  Ground_2 : Ground
  Out1 : Outport  OutDataTypeStr=Bus: CmptmtAirTEstimdExtd  PortDimensions=1
  wires:
  Ground_2/1 -> Out1/1

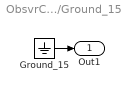
== system ObsvrCmptmtT/ObsvrCmptmtT_Runnable_sys/Ground_15 ==
  Ground_15 : Ground
  Out1 : Outport  OutDataTypeStr=Bus: CmptmtAirTEstimdExtd  PortDimensions=1
  wires:
  Ground_15/1 -> Out1/1

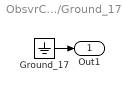
== system ObsvrCmptmtT/ObsvrCmptmtT_Runnable_sys/Ground_17 ==
  Ground_17 : Ground
  Out1 : Outport  OutDataTypeStr=Bus: MtrlSnsrT  PortDimensions=1
  wires:
  Ground_17/1 -> Out1/1

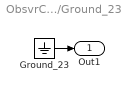
== system ObsvrCmptmtT/ObsvrCmptmtT_Runnable_sys/Ground_23 ==
  Ground_23 : Ground
  Out1 : Outport  OutDataTypeStr=Bus: MemRecForObsvrCmptmT  PortDimensions=1
  wires:
  Ground_23/1 -> Out1/1
